simulink library "lib_all"  (Simulink 7.6)
settings: Solver=ode45  SolverMode=SingleTasking  StartTime=0.0  StopTime=10.0  RelTol=1e-3
values from neighbouring files (symbols this model uses):
  map_lib_03 = [6.8679 7.0159 7.4571 8.1849 9.1892 10.456 11.97 13.71 15.655 17.781 20.063 22.474 ... (803 elements, 73x11)]    [scr_simdata.mat]    (also in scr_simdata_all.mat)
  map_lib_06 = [2.5784 2.6727 2.9588 3.4453 4.1444 5.068 6.226 7.6242 9.2634 11.139 13.24 15.552 ... (803 elements, 73x11)]    [scr_simdata.mat]    (also in scr_simdata_all.mat)
  map_lib_09 = [0.0025784 0.0026727 0.0029588 0.0034453 0.0041444 0.005068 0.006226 0.0076242 0.0092634 0.011139 0.01324 0.015552 ... (803 elements, 73x11)]    [scr_simdata.mat]    (also in scr_simdata_all.mat)
  map_lib_10 = [2.74 2.75 2.76 2.77 2.78 2.79 2.8 2.81 2.82 2.83 2.84 2.85 ... (81 elements, 1x81)]    [scr_simdata.mat]    (also in scr_simdata_all.mat)
  map_lib_11 = [1.1983 1.2027 1.207 1.2113 1.2156 1.2198 1.2241 1.2283 1.2326 1.2368 1.241 1.2451 ... (81 elements, 1x81)]    [scr_simdata.mat]    (also in scr_simdata_all.mat)
  map_lib_12 = [2.74 2.75 2.76 2.77 2.78 2.79 2.8 2.81 2.82 2.83 2.84 2.85 ... (81 elements, 1x81)]    [scr_simdata.mat]    (also in scr_simdata_all.mat)
  map_lib_13 = [1.74 1.75 1.76 1.77 1.78 1.79 1.8 1.81 1.82 1.83 1.84 1.85 ... (81 elements, 1x81)]    [scr_simdata.mat]    (also in scr_simdata_all.mat)
  map_lib_16 = [0.00048828125 1.6159 1.8962 2.0821 2.2251 2.3426 2.4433 2.5318 2.611 2.683 2.749 2.8101 ... (1419 elements, 129x11)]    [scr_simdata.mat]    (also in scr_simdata_all.mat)
  map_mr2_01 = [0 800 1600 2400 3200 4000]    [scr_simdata.mat]    (also in scr_simdata_all.mat)
  map_mr2_02 = [1.32 1.32 1.33 1.34 1.36 1.38]    [scr_simdata.mat]    (also in scr_simdata_all.mat)
  map_mr2_03 = [0 800 1600 2400 3200 4000]    [scr_simdata.mat]    (also in scr_simdata_all.mat)
  map_mr2_04 = [1.32 1.32 1.33 1.34 1.36 1.38]    [scr_simdata.mat]    (also in scr_simdata_all.mat)
  map_mr2_05 = [0 800 1600 2400 3200 4000]    [scr_simdata.mat]    (also in scr_simdata_all.mat)
  map_mr2_06 = [1.32 1.32 1.33 1.34 1.36 1.38]    [scr_simdata.mat]    (also in scr_simdata_all.mat)
  Parameter1 = 6    [scr_simdata.mat]    (also in scr_simdata_all.mat)
  Parameter101 = 30    [scr_simdata.mat]    (also in scr_simdata_all.mat)
  Parameter102 = 12    [scr_simdata.mat]    (also in scr_simdata_all.mat)
  Parameter103 = -240    [scr_simdata.mat]    (also in scr_simdata_all.mat)
  Parameter108 = 48    [scr_simdata.mat]    (also in scr_simdata_all.mat)
  Parameter120 = 16    [scr_simdata.mat]    (also in scr_simdata_all.mat)
  Parameter121 = 87    [scr_simdata.mat]    (also in scr_simdata_all.mat)
  Parameter123 = 78    [scr_simdata.mat]    (also in scr_simdata_all.mat)
  Parameter124 = 60    [scr_simdata.mat]    (also in scr_simdata_all.mat)
  Parameter125 = -480    [scr_simdata.mat]    (also in scr_simdata_all.mat)
  Parameter130 = 96    [scr_simdata.mat]    (also in scr_simdata_all.mat)
  Parameter131 = 3    [scr_simdata.mat]    (also in scr_simdata_all.mat)
  Parameter144 = 3    [scr_simdata.mat]    (also in scr_simdata_all.mat)
  Parameter18 = 128    [scr_simdata.mat]    (also in scr_simdata_all.mat)
  Parameter19 = 16    [scr_simdata.mat]    (also in scr_simdata_all.mat)
  Parameter2 = 12    [scr_simdata.mat]    (also in scr_simdata_all.mat)
  Parameter20 = 7    [scr_simdata.mat]    (also in scr_simdata_all.mat)
  Parameter21 = 4    [scr_simdata.mat]    (also in scr_simdata_all.mat)
  Parameter3 = 18    [scr_simdata.mat]    (also in scr_simdata_all.mat)
  Parameter32 = 1    [scr_simdata.mat]    (also in scr_simdata_all.mat)
  Parameter34 = 24    [scr_simdata.mat]    (also in scr_simdata_all.mat)
  Parameter4 = 138    [scr_simdata.mat]    (also in scr_simdata_all.mat)
  Parameter42 = 48    [scr_simdata.mat]    (also in scr_simdata_all.mat)
  Parameter5 = 135    [scr_simdata.mat]    (also in scr_simdata_all.mat)
  Parameter55 = 0    [scr_simdata.mat]    (also in scr_simdata_all.mat)
  Parameter56 = 0    [scr_simdata.mat]    (also in scr_simdata_all.mat)
  Parameter57 = 1    [scr_simdata.mat]    (also in scr_simdata_all.mat)
  Parameter58 = 1    [scr_simdata.mat]    (also in scr_simdata_all.mat)
  Parameter59 = 2    [scr_simdata.mat]    (also in scr_simdata_all.mat)
  Parameter6 = 132    [scr_simdata.mat]    (also in scr_simdata_all.mat)
  Parameter60 = 2    [scr_simdata.mat]    (also in scr_simdata_all.mat)
  Parameter63 = 144    [scr_simdata.mat]    (also in scr_simdata_all.mat)
  Parameter7 = 126    [scr_simdata.mat]    (also in scr_simdata_all.mat)
  Parameter76 = 64    [scr_simdata.mat]    (also in scr_simdata_all.mat)
  Parameter77 = 135    [scr_simdata.mat]    (also in scr_simdata_all.mat)
  Parameter79 = 126    [scr_simdata.mat]    (also in scr_simdata_all.mat)
  Parameter80 = 108    [scr_simdata.mat]    (also in scr_simdata_all.mat)
  Parameter81 = 0    [scr_simdata.mat]    (also in scr_simdata_all.mat)
  Parameter86 = 0    [scr_simdata.mat]    (also in scr_simdata_all.mat)
  Parameter98 = 112    [scr_simdata.mat]    (also in scr_simdata_all.mat)
  Parameter99 = 39    [scr_simdata.mat]    (also in scr_simdata_all.mat)
undefined symbols (not defined in the file or in its folder): cylvolkp_hih_map2h, cylvolkp_map2h, pk1k_map2h
libraries: simulink x2
(part 36 of 36)

== system lib_all/SubSystem7/SubSystem9/SubSystem13/SubSystem67/SubSystem76/SubSystem90/SubSystem96/SubSystem98 ==
  note: ***編集禁止***
  element : Inport
  Inside_DSR : DataStoreRead  DataStoreName=DSM6
  Selector : Selector  IndexOptions=Index vector (port)  Indices=[1 3]  OutputSizes=1
  Output : Outport
  wires:
  Selector/1 -> Output/1
  element/1 -> Selector/2
  Inside_DSR/1 -> Selector/1

== system lib_all/SubSystem7/SubSystem9/SubSystem13/SubSystem67/SubSystem76/SubSystem90/SubSystem96/SubSystem99 ==
  note: ***編集禁止***
  element : Inport
  Inside_DSR : DataStoreRead  DataStoreName=DSM19
  Selector : Selector  IndexOptions=Index vector (port)  Indices=[1 3]  OutputSizes=1
  Output : Outport
  wires:
  Inside_DSR/1 -> Selector/1
  element/1 -> Selector/2
  Selector/1 -> Output/1

== stateflow chart SubSystem176/SubSystem303/SubSystem305/SubSystem306/For_loop ==
  note: for(i1 = 0; i1 < N_Loop; i1++) {
      |   処理
      | }
  (default) -> (junction 4) : {
      |  idx = 1;
      |  i1 = 0;
      | }
  (junction 4) -> (junction 5)
  (junction 5) -> (junction 6) [order 1] : [i1 < N_Loop]
  (junction 6) -> (junction 7) : {
      |  function_call;
      |  idx = idx + 1;
      | }
  (junction 5) -> (junction 8) [order 2]
  (junction 7) -> (junction 4) : {i1++;}
  data: N_Loop input double; i1 local int32; idx output int32
  events: function_call output FUNCTION_CALL_EVENT

== stateflow chart SubSystem176/SubSystem303/SubSystem305/SubSystem308/SubSystem313/SubSystem316/For_loop ==
  note: for(i1 = 0; i1 < N_Loop; i1++) {
      |   処理
      | }
  (default) -> (junction 22) : {
      |  idx = 1;
      |  i1 = 0;
      | }
  (junction 22) -> (junction 23)
  (junction 23) -> (junction 24) [order 1] : [i1 < N_Loop]
  (junction 24) -> (junction 25) : {
      |  function_call;
      |  idx = idx + 1;
      | }
  (junction 23) -> (junction 26) [order 2]
  (junction 25) -> (junction 22) : {i1++;}
  data: N_Loop input double; i1 local int32; idx output int32
  events: function_call output FUNCTION_CALL_EVENT

== stateflow chart SubSystem176/SubSystem329/SubSystem332/SubSystem333/For_loop ==
  note: for(i1 = 0; i1 < N_Loop; i1++) {
      |   処理
      | }
  (default) -> (junction 40) : {
      |  idx = 1;
      |  i1 = 0;
      | }
  (junction 40) -> (junction 41)
  (junction 41) -> (junction 42) [order 1] : [i1 < N_Loop]
  (junction 42) -> (junction 43) : {
      |  function_call;
      |  idx = idx + 1;
      | }
  (junction 41) -> (junction 44) [order 2]
  (junction 43) -> (junction 40) : {i1++;}
  data: N_Loop input double; i1 local int32; idx output int32
  events: function_call output FUNCTION_CALL_EVENT

== stateflow chart SubSystem176/SubSystem329/SubSystem332/SubSystem335/For_loop ==
  note: for(i1 = 0; i1 < N_Loop; i1++) {
      |   処理
      | }
  (default) -> (junction 58) : {
      |  idx = 1;
      |  i1 = 0;
      | }
  (junction 58) -> (junction 59)
  (junction 59) -> (junction 60) [order 1] : [i1 < N_Loop]
  (junction 60) -> (junction 61) : {
      |  function_call;
      |  idx = idx + 1;
      | }
  (junction 59) -> (junction 62) [order 2]
  (junction 61) -> (junction 58) : {i1++;}
  data: N_Loop input double; i1 local int32; idx output int32
  events: function_call output FUNCTION_CALL_EVENT

== stateflow chart SubSystem1770/SubSystem1774/SubSystem1778/SubSystem1779/SingleForIterator/For_loop ==
  note: for(i1 = 0; i1 < N_Loop; i1++) {
      |   処理
      | }
  (default) -> (junction 76) : {
      |  idx = 1;
      |  i1 = 0;
      | }
  (junction 76) -> (junction 77)
  (junction 77) -> (junction 78) [order 1] : [i1 < N_Loop]
  (junction 78) -> (junction 79) : {
      |  function_call;
      |  idx = idx + 1;
      | }
  (junction 77) -> (junction 80) [order 2]
  (junction 79) -> (junction 76) : {i1++;}
  data: N_Loop input double; i1 local int32; idx output int32
  events: function_call output FUNCTION_CALL_EVENT

== stateflow chart SubSystem1770/SubSystem1774/SubSystem1791/SubSystem1792/SubSystem1794/For_loop ==
  note: for(i1 = 0; i1 < N_Loop; i1++) {
      |   処理
      | }
  (default) -> (junction 94) : {
      |  idx = 1;
      |  i1 = 0;
      | }
  (junction 94) -> (junction 95)
  (junction 95) -> (junction 96) [order 1] : [i1 < N_Loop]
  (junction 96) -> (junction 97) : {
      |  function_call;
      |  idx = idx + 1;
      | }
  (junction 95) -> (junction 98) [order 2]
  (junction 97) -> (junction 94) : {i1++;}
  data: N_Loop input double; i1 local int32; idx output int32
  events: function_call output FUNCTION_CALL_EVENT

== stateflow chart SubSystem1770/SubSystem1774/SubSystem1804/SubSystem1805/SubSystem1807/For_loop ==
  note: for(i1 = 0; i1 < N_Loop; i1++) {
      |   処理
      | }
  (default) -> (junction 112) : {
      |  idx = 1;
      |  i1 = 0;
      | }
  (junction 112) -> (junction 113)
  (junction 113) -> (junction 114) [order 1] : [i1 < N_Loop]
  (junction 114) -> (junction 115) : {
      |  function_call;
      |  idx = idx + 1;
      | }
  (junction 113) -> (junction 116) [order 2]
  (junction 115) -> (junction 112) : {i1++;}
  data: N_Loop input double; i1 local int32; idx output int32
  events: function_call output FUNCTION_CALL_EVENT

== stateflow chart SubSystem1770/SubSystem1817/SubSystem1821/SubSystem1822/SubSystem1824/For_loop ==
  note: for(i1 = 0; i1 < N_Loop; i1++) {
      |   処理
      | }
  (default) -> (junction 130) : {
      |  idx = 1;
      |  i1 = 0;
      | }
  (junction 130) -> (junction 131)
  (junction 131) -> (junction 132) [order 1] : [i1 < N_Loop]
  (junction 132) -> (junction 133) : {
      |  function_call;
      |  idx = idx + 1;
      | }
  (junction 131) -> (junction 134) [order 2]
  (junction 133) -> (junction 130) : {i1++;}
  data: N_Loop input double; i1 local int32; idx output int32
  events: function_call output FUNCTION_CALL_EVENT

== stateflow chart SubSystem1770/SubSystem1817/SubSystem1834/SubSystem1835/SubSystem1837/For_loop ==
  note: for(i1 = 0; i1 < N_Loop; i1++) {
      |   処理
      | }
  (default) -> (junction 148) : {
      |  idx = 1;
      |  i1 = 0;
      | }
  (junction 148) -> (junction 149)
  (junction 149) -> (junction 150) [order 1] : [i1 < N_Loop]
  (junction 150) -> (junction 151) : {
      |  function_call;
      |  idx = idx + 1;
      | }
  (junction 149) -> (junction 152) [order 2]
  (junction 151) -> (junction 148) : {i1++;}
  data: N_Loop input double; i1 local int32; idx output int32
  events: function_call output FUNCTION_CALL_EVENT

== stateflow chart SubSystem1770/SubSystem1817/SubSystem1847/SubSystem1848/SubSystem1850/For_loop ==
  note: for(i1 = 0; i1 < N_Loop; i1++) {
      |   処理
      | }
  (default) -> (junction 166) : {
      |  idx = 1;
      |  i1 = 0;
      | }
  (junction 166) -> (junction 167)
  (junction 167) -> (junction 168) [order 1] : [i1 < N_Loop]
  (junction 168) -> (junction 169) : {
      |  function_call;
      |  idx = idx + 1;
      | }
  (junction 167) -> (junction 170) [order 2]
  (junction 169) -> (junction 166) : {i1++;}
  data: N_Loop input double; i1 local int32; idx output int32
  events: function_call output FUNCTION_CALL_EVENT

== stateflow chart SubSystem1860/SubSystem1862/For_loop ==
  note: for(i1 = 0; i1 < N_Loop; i1++) {
      |   処理
      | }
  (default) -> (junction 184) : {
      |  idx = 1;
      |  i1 = 0;
      | }
  (junction 184) -> (junction 185)
  (junction 185) -> (junction 186) [order 1] : [i1 < N_Loop]
  (junction 186) -> (junction 187) : {
      |  function_call;
      |  idx = idx + 1;
      | }
  (junction 185) -> (junction 188) [order 2]
  (junction 187) -> (junction 184) : {i1++;}
  data: N_Loop input double; i1 local int32; idx output int32
  events: function_call output FUNCTION_CALL_EVENT

== stateflow chart SubSystem347/SubSystem477/SubSystem479/SubSystem480/For_loop ==
  note: for(i1 = 0; i1 < N_Loop; i1++) {
      |   処理
      | }
  (default) -> (junction 202) : {
      |  idx = 1;
      |  i1 = 0;
      | }
  (junction 202) -> (junction 203)
  (junction 203) -> (junction 204) [order 1] : [i1 < N_Loop]
  (junction 204) -> (junction 205) : {
      |  function_call;
      |  idx = idx + 1;
      | }
  (junction 203) -> (junction 206) [order 2]
  (junction 205) -> (junction 202) : {i1++;}
  data: N_Loop input double; i1 local int32; idx output int32
  events: function_call output FUNCTION_CALL_EVENT

== stateflow chart SubSystem347/SubSystem477/SubSystem479/SubSystem482/SubSystem487/SubSystem490/For_loop ==
  note: for(i1 = 0; i1 < N_Loop; i1++) {
      |   処理
      | }
  (default) -> (junction 220) : {
      |  idx = 1;
      |  i1 = 0;
      | }
  (junction 220) -> (junction 221)
  (junction 221) -> (junction 222) [order 1] : [i1 < N_Loop]
  (junction 222) -> (junction 223) : {
      |  function_call;
      |  idx = idx + 1;
      | }
  (junction 221) -> (junction 224) [order 2]
  (junction 223) -> (junction 220) : {i1++;}
  data: N_Loop input double; i1 local int32; idx output int32
  events: function_call output FUNCTION_CALL_EVENT

== stateflow chart SubSystem347/SubSystem503/SubSystem506/SubSystem507/For_loop ==
  note: for(i1 = 0; i1 < N_Loop; i1++) {
      |   処理
      | }
  (default) -> (junction 238) : {
      |  idx = 1;
      |  i1 = 0;
      | }
  (junction 238) -> (junction 239)
  (junction 239) -> (junction 240) [order 1] : [i1 < N_Loop]
  (junction 240) -> (junction 241) : {
      |  function_call;
      |  idx = idx + 1;
      | }
  (junction 239) -> (junction 242) [order 2]
  (junction 241) -> (junction 238) : {i1++;}
  data: N_Loop input double; i1 local int32; idx output int32
  events: function_call output FUNCTION_CALL_EVENT

== stateflow chart SubSystem347/SubSystem503/SubSystem506/SubSystem509/For_loop ==
  note: for(i1 = 0; i1 < N_Loop; i1++) {
      |   処理
      | }
  (default) -> (junction 256) : {
      |  idx = 1;
      |  i1 = 0;
      | }
  (junction 256) -> (junction 257)
  (junction 257) -> (junction 258) [order 1] : [i1 < N_Loop]
  (junction 258) -> (junction 259) : {
      |  function_call;
      |  idx = idx + 1;
      | }
  (junction 257) -> (junction 260) [order 2]
  (junction 259) -> (junction 256) : {i1++;}
  data: N_Loop input double; i1 local int32; idx output int32
  events: function_call output FUNCTION_CALL_EVENT

== stateflow chart SubSystem7/SubSystem134/SubSystem136/SubSystem137/For_loop ==
  note: for(i1 = 0; i1 < N_Loop; i1++) {
      |   処理
      | }
  (default) -> (junction 274) : {
      |  idx = 1;
      |  i1 = 0;
      | }
  (junction 274) -> (junction 275)
  (junction 275) -> (junction 276) [order 1] : [i1 < N_Loop]
  (junction 276) -> (junction 277) : {
      |  function_call;
      |  idx = idx + 1;
      | }
  (junction 275) -> (junction 278) [order 2]
  (junction 277) -> (junction 274) : {i1++;}
  data: N_Loop input double; i1 local int32; idx output int32
  events: function_call output FUNCTION_CALL_EVENT

== stateflow chart SubSystem7/SubSystem134/SubSystem136/SubSystem139/SubSystem144/SubSystem147/For_loop ==
  note: for(i1 = 0; i1 < N_Loop; i1++) {
      |   処理
      | }
  (default) -> (junction 292) : {
      |  idx = 1;
      |  i1 = 0;
      | }
  (junction 292) -> (junction 293)
  (junction 293) -> (junction 294) [order 1] : [i1 < N_Loop]
  (junction 294) -> (junction 295) : {
      |  function_call;
      |  idx = idx + 1;
      | }
  (junction 293) -> (junction 296) [order 2]
  (junction 295) -> (junction 292) : {i1++;}
  data: N_Loop input double; i1 local int32; idx output int32
  events: function_call output FUNCTION_CALL_EVENT

== stateflow chart SubSystem7/SubSystem161/SubSystem164/SubSystem165/For_loop ==
  note: for(i1 = 0; i1 < N_Loop; i1++) {
      |   処理
      | }
  (default) -> (junction 310) : {
      |  idx = 1;
      |  i1 = 0;
      | }
  (junction 310) -> (junction 311)
  (junction 311) -> (junction 312) [order 1] : [i1 < N_Loop]
  (junction 312) -> (junction 313) : {
      |  function_call;
      |  idx = idx + 1;
      | }
  (junction 311) -> (junction 314) [order 2]
  (junction 313) -> (junction 310) : {i1++;}
  data: N_Loop input double; i1 local int32; idx output int32
  events: function_call output FUNCTION_CALL_EVENT

== stateflow chart SubSystem7/SubSystem161/SubSystem164/SubSystem167/For_loop ==
  note: for(i1 = 0; i1 < N_Loop; i1++) {
      |   処理
      | }
  (default) -> (junction 328) : {
      |  idx = 1;
      |  i1 = 0;
      | }
  (junction 328) -> (junction 329)
  (junction 329) -> (junction 330) [order 1] : [i1 < N_Loop]
  (junction 330) -> (junction 331) : {
      |  function_call;
      |  idx = idx + 1;
      | }
  (junction 329) -> (junction 332) [order 2]
  (junction 331) -> (junction 328) : {i1++;}
  data: N_Loop input double; i1 local int32; idx output int32
  events: function_call output FUNCTION_CALL_EVENT

== stateflow chart SubSystem959/SubSystem961/For_loop ==
  note: for(i1 = 0; i1 < N_Loop; i1++) {
      |   処理
      | }
  (default) -> (junction 346) : {
      |  idx = 1;
      |  i1 = 0;
      | }
  (junction 346) -> (junction 347)
  (junction 347) -> (junction 348) [order 1] : [i1 < N_Loop]
  (junction 348) -> (junction 349) : {
      |  function_call;
      |  idx = idx + 1;
      | }
  (junction 347) -> (junction 350) [order 2]
  (junction 349) -> (junction 346) : {i1++;}
  data: N_Loop input double; i1 local int32; idx output int32
  events: function_call output FUNCTION_CALL_EVENT
